simulink model "CCMHfaMotor"  (R2022b) (continued)
== system CCMHfaMotor/HfaMotor_Runnable_sys == (continued)
  ReHvacMotUrgcUnlck_ReHvacMotUrgcUnlck_write : Outport  Port=59
      settings: EnsureOutportIsVirtual=on
  RHFAScheTableReq_Val_write : Outport  Port=60
      settings: EnsureOutportIsVirtual=on
  WtrMotAdr_WtrMotAdr_write : Outport  Port=61
      settings: EnsureOutportIsVirtual=on
  WtrMotBlockCmd_WtrMotBlockCmd_write : Outport  Port=62
      settings: EnsureOutportIsVirtual=on
  WtrMotClearReq_WtrMotClearReq_write : Outport  Port=63
      settings: EnsureOutportIsVirtual=on
  WtrMotCoilPwrOn_WtrMotCoilPwrOn_write : Outport  Port=64
      settings: EnsureOutportIsVirtual=on
  WtrMotDir_WtrMotDir_write : Outport  Port=65
      settings: EnsureOutportIsVirtual=on
  WtrMotMode_WtrMotMode_write : Outport  Port=66
      settings: EnsureOutportIsVirtual=on
  WtrMotPsonAvl_WtrMotPsonAvl_write : Outport  Port=67
      settings: EnsureOutportIsVirtual=on
  WtrMotSaveData_WtrMotSaveData_write : Outport  Port=68
      settings: EnsureOutportIsVirtual=on
  WtrMotSpdSet_WtrMotSpdSet_write : Outport  Port=69
      settings: EnsureOutportIsVirtual=on
  WtrMotStrtPson_WtrMotStrtPson_write : Outport  Port=70
      settings: EnsureOutportIsVirtual=on
  WtrMotTargtPson_WtrMotTargtPson_write : Outport  Port=71
      settings: EnsureOutportIsVirtual=on
  WtrMotUrgcPson_WtrMotUrgcPson_write : Outport  Port=72
      settings: EnsureOutportIsVirtual=on
  WtrMotUrgcUnlck_WtrMotUrgcUnlck_write : Outport  Port=73
      settings: EnsureOutportIsVirtual=on
  HfaMotAdr_HfaMotAdr_write : Outport  Port=74
      settings: EnsureOutportIsVirtual=on
  HfaMotBlockCmd_HfaMotBlockCmd_write : Outport  Port=75
      settings: EnsureOutportIsVirtual=on
  HfaMotClearReq_HfaMotClearReq_write : Outport  Port=76
      settings: EnsureOutportIsVirtual=on
  HfaMotCoilPwrOn_HfaMotCoilPwrOn_write : Outport  Port=77
      settings: EnsureOutportIsVirtual=on
  HfaMotDir_HfaMotDir_write : Outport  Port=78
      settings: EnsureOutportIsVirtual=on
  HfaMotMode_HfaMotMode_write : Outport  Port=79
      settings: EnsureOutportIsVirtual=on
  HfaMotPsonAvl_HfaMotPsonAvl_write : Outport  Port=80
      settings: EnsureOutportIsVirtual=on
  HfaMotSaveData_HfaMotSaveData_write : Outport  Port=81
      settings: EnsureOutportIsVirtual=on
  HfaMotSpdSet_HfaMotSpdSet_write : Outport  Port=82
      settings: EnsureOutportIsVirtual=on
  HfaMotStrtPson_HfaMotStrtPson_write : Outport  Port=83
      settings: EnsureOutportIsVirtual=on
  HfaMotTargtPson_HfaMotTargtPson_write : Outport  Port=84
      settings: EnsureOutportIsVirtual=on
  HfaMotUrgcPson_HfaMotUrgcPson_write : Outport  Port=85
      settings: EnsureOutportIsVirtual=on
  HfaMotUrgcUnlck_HfaMotUrgcUnlck_write : Outport  Port=86
      settings: EnsureOutportIsVirtual=on
  wires:
  AcRfrPrgSafety_AcRfrPrgSafety_read/1 -> Terminator_1/1
  ActRfrIdeGroup_ActRfrIdeGroup_read/1 -> Terminator_2/1
  ActRfrIdeItem_ActRfrIdeItem_read/1 -> Terminator_3/1
  ActRfrIdePN_ActRfrIdePN_read/1 -> Terminator_4/1
  ActRfrIdeSupplier_ActRfrIdeSupplier_read/1 -> Terminator_5/1
  ActRfrIdeVers_ActRfrIdeVers_read/1 -> Terminator_6/1
  ActRfrPrgDiret_ActRfrPrgDiret_read/1 -> Terminator_7/1
  ActRfrPrgGroup_ActRfrPrgGroup_read/1 -> Terminator_8/1
  ActRfrPrgItem_ActRfrPrgItem_read/1 -> Terminator_9/1
  ActRfrPrgPrgGroup_ActRfrPrgPrgGroup_read/1 -> Terminator_10/1
  ActRfrPrgPrgItem_ActRfrPrgPrgItem_read/1 -> Terminator_11/1
  ActRfrPrgRef_ActRfrPrgRef_read/1 -> Terminator_12/1
  ActRfrPrgSafetyEnable_ActRfrPrgSafetyEnable_read/1 -> Terminator_13/1
  ActRfrStaCPos_ActRfrStaCPos_read/1 -> Terminator_14/1
  ActRfrStaEmgyRun_ActRfrStaEmgyRun_read/1 -> Terminator_15/1
  ActRfrStaErr_ActRfrStaErr_read/1 -> Terminator_16/1
  ActRfrStaGroup_ActRfrStaGroup_read/1 -> Terminator_17/1
  ActRfrStaItem_ActRfrStaItem_read/1 -> Terminator_18/1
  ActRfrStaMod_ActRfrStaMod_read/1 -> Terminator_19/1
  ActRfrStaReset_ActRfrStaReset_read/1 -> Terminator_20/1
  ActRfrStaStd_ActRfrStaStd_read/1 -> Terminator_21/1
  BatSysBtrMSts_BatSysBtrMSts_read/1 -> Terminator_22/1
  HfaCcmSlaveRespB0Lin4_HfaCcmSlaveRespB0Lin4_read/1 -> Terminator_23/1
  HfaCcmSlaveRespB1Lin4_HfaCcmSlaveRespB1Lin4_read/1 -> Terminator_24/1
  HfaCcmSlaveRespB2Lin4_HfaCcmSlaveRespB2Lin4_read/1 -> Terminator_25/1
  HfaCcmSlaveRespB3Lin4_HfaCcmSlaveRespB3Lin4_read/1 -> Terminator_26/1
  HfaCcmSlaveRespB4Lin4_HfaCcmSlaveRespB4Lin4_read/1 -> Terminator_27/1
  HfaCcmSlaveRespB5Lin4_HfaCcmSlaveRespB5Lin4_read/1 -> Terminator_28/1
  HfaCcmSlaveRespB6Lin4_HfaCcmSlaveRespB6Lin4_read/1 -> Terminator_29/1
  HfaCcmSlaveRespB7Lin4_HfaCcmSlaveRespB7Lin4_read/1 -> Terminator_30/1
  HmiElecAirSwngReq_HmiElecAirSwngReq_read/1 -> Terminator_31/1
  HvacCfgForClimaCtrl_HvacCfgForHvacFrontBlowerType_read/1 -> Terminator_32/1
  HvacCfgForClimaCtrl_HvacCfgForHvacMiscExistSts_read/1 -> Terminator_33/1
  HvacCfgForClimaCtrl_HvacCfgForHvacMotorExistSts_read/1 -> Terminator_34/1
  HvacCfgForClimaCtrl_HvacCfgForHvacSensorExistSts_read/1 -> Terminator_35/1
  SbcPwrOn_SbcPwrOn_read/1 -> Terminator_36/1
  SbcWakeupSta_SbcWakeupSta_read/1 -> Terminator_37/1
  SysServCarCfgSts_SysServCarID_read/1 -> Terminator_38/1
  SysServCarCfgSts_SysServCCPAQSSts_read/1 -> Terminator_39/1
  SysServCarCfgSts_SysServCCPHEXSensorSts_read/1 -> Terminator_40/1
  SysServCarCfgSts_SysServCCPLin2AFUSts_read/1 -> Terminator_41/1
  SysServCarCfgSts_SysServCCPLin2AIUSts_read/1 -> Terminator_42/1
  SysServCarCfgSts_SysServCCPLin2CDSSts_read/1 -> Terminator_43/1
  SysServCarCfgSts_SysServCCPLin2PMSISts_read/1 -> Terminator_44/1
  SysServCarCfgSts_SysServCCPLin3VGASts_read/1 -> Terminator_45/1
  SysServCarCfgSts_SysServDUAirVentSnrSts_read/1 -> Terminator_46/1
  SysServCarCfgSts_SysServDUBlowerPwmSignSts_read/1 -> Terminator_47/1
  SysServCarCfgSts_SysServDUBlowerPwmSts_read/1 -> Terminator_48/1
  SysServCarCfgSts_SysServDUDCDefrostSts_read/1 -> Terminator_49/1
  SysServCarCfgSts_SysServDUDCModeSts_read/1 -> Terminator_50/1
  SysServCarCfgSts_SysServDUDCRecSts_read/1 -> Terminator_51/1
  SysServCarCfgSts_SysServDUDCSModeSts_read/1 -> Terminator_52/1
  SysServCarCfgSts_SysServDUDCSTLSts_read/1 -> Terminator_53/1
  SysServCarCfgSts_SysServDUDCTLSts_read/1 -> Terminator_54/1
  SysServCarCfgSts_SysServDUDCTRSts_read/1 -> Terminator_55/1
  SysServCarCfgSts_SysServDUFLFootSts_read/1 -> Terminator_56/1
  SysServCarCfgSts_SysServDUFLVentSts_read/1 -> Terminator_57/1
  SysServCarCfgSts_SysServDUFRFootSts_read/1 -> Terminator_58/1
  SysServCarCfgSts_SysServDUFRVentSts_read/1 -> Terminator_59/1
  SysServCarCfgSts_SysServDUIncarMotorSts_read/1 -> Terminator_60/1
  SysServCarCfgSts_SysServDULin1HBMFSts_read/1 -> Terminator_61/1
  SysServCarCfgSts_SysServDULin4HFAFSts_read/1 -> Terminator_62/1
  SysServCarCfgSts_SysServDUSFLFootSts_read/1 -> Terminator_63/1
  SysServCarCfgSts_SysServDUSFLVentSts_read/1 -> Terminator_64/1
  SysServCarCfgSts_SysServDUSFRFootSts_read/1 -> Terminator_65/1
  SysServCarCfgSts_SysServDUSFRVentSts_read/1 -> Terminator_66/1
  SysServCarCfgSts_SysServDUSnsrForFCmptmtTSts_read/1 -> Terminator_67/1
  SysServCarCfgSts_SysServDUSnsrForRair_read/1 -> Terminator_68/1
  SysServCarCfgSts_SysServDUSnsrForRCmptmtTSts_read/1 -> Terminator_69/1
  BatLoadBtrMSts_BatLoadBtrMSts_read/1 -> Terminator_70/1
  HfaMotSetPowerOnInit_PowerOnInit_read/1 -> Terminator_71/1
  VehModMngtGlbSafe1_VehModMngtGlbSafe1_read/1 -> Terminator_72/1
  HfaActr01ActPson_HfaActr01ActPson_read/1 -> Terminator_73/1
  HfaActr01ActPson_HfaActr01ActPson_IsUpdated_read/1 -> Terminator_74/1
  HfaActr01BlckInd_HfaActr01BlckInd_read/1 -> Terminator_75/1
  HfaActr01BlckSts_HfaActr01BlckSts_read/1 -> Terminator_76/1
  HfaActr01BusAdr_HfaActr01BusAdr_read/1 -> Terminator_77/1
  HfaActr01CoilSts_HfaActr01CoilSts_read/1 -> Terminator_78/1
  HfaActr01DrvDir_HfaActr01DrvDir_read/1 -> Terminator_79/1
  HfaActr01ElecErr_HfaActr01ElecErr_read/1 -> Terminator_80/1
  HfaActr01Mode_HfaActr01Mode_read/1 -> Terminator_81/1
  HfaActr01MovDir_HfaActr01MovDir_read/1 -> Terminator_82/1
  HfaActr01OvrTempErr_HfaActr01OvrTempErr_read/1 -> Terminator_83/1
  HfaActr01PsonSts_HfaActr01PsonSts_read/1 -> Terminator_84/1
  HfaActr01ResetSts_HfaActr01ResetSts_read/1 -> Terminator_85/1
  HfaActr01SpclFct_HfaActr01SpclFct_read/1 -> Terminator_86/1
  HfaActr01SpdSts_HfaActr01SpdSts_read/1 -> Terminator_87/1
  HfaActr01TrqSts_HfaActr01TrqSts_read/1 -> Terminator_88/1
  HfaActr01UrgcExist_HfaActr01UrgcExist_read/1 -> Terminator_89/1
  HfaActr01UrgcPson_HfaActr01UrgcPson_read/1 -> Terminator_90/1
  HfaActr01UrgcSts_HfaActr01UrgcSts_read/1 -> Terminator_91/1
  HfaActr01VoltErr_HfaActr01VoltErr_read/1 -> Terminator_92/1
  HfaActr02ActPson_HfaActr02ActPson_read/1 -> Terminator_93/1
  HfaActr02ActPson_HfaActr02ActPson_IsUpdated_read/1 -> Terminator_94/1
  HfaActr02BlckInd_HfaActr02BlckInd_read/1 -> Terminator_95/1
  HfaActr02BlckSts_HfaActr02BlckSts_read/1 -> Terminator_96/1
  HfaActr02BusAdr_HfaActr02BusAdr_read/1 -> Terminator_97/1
  HfaActr02CoilSts_HfaActr02CoilSts_read/1 -> Terminator_98/1
  HfaActr02DrvDir_HfaActr02DrvDir_read/1 -> Terminator_99/1
  HfaActr02ElecErr_HfaActr02ElecErr_read/1 -> Terminator_100/1
  HfaActr02Mode_HfaActr02Mode_read/1 -> Terminator_101/1
  HfaActr02MovDir_HfaActr02MovDir_read/1 -> Terminator_102/1
  HfaActr02OvrTempErr_HfaActr02OvrTempErr_read/1 -> Terminator_103/1
  HfaActr02PsonSts_HfaActr02PsonSts_read/1 -> Terminator_104/1
  HfaActr02ResetSts_HfaActr02ResetSts_read/1 -> Terminator_105/1
  HfaActr02SpclFct_HfaActr02SpclFct_read/1 -> Terminator_106/1
  HfaActr02SpdSts_HfaActr02SpdSts_read/1 -> Terminator_107/1
  HfaActr02TrqSts_HfaActr02TrqSts_read/1 -> Terminator_108/1
  HfaActr02UrgcExist_HfaActr02UrgcExist_read/1 -> Terminator_109/1
  HfaActr02UrgcPson_HfaActr02UrgcPson_read/1 -> Terminator_110/1
  HfaActr02UrgcSts_HfaActr02UrgcSts_read/1 -> Terminator_111/1
  HfaActr02VoltErr_HfaActr02VoltErr_read/1 -> Terminator_112/1
  HfaActr03ActPson_HfaActr03ActPson_read/1 -> Terminator_113/1
  HfaActr03ActPson_HfaActr03ActPson_IsUpdated_read/1 -> Terminator_114/1
  HfaActr03BlckInd_HfaActr03BlckInd_read/1 -> Terminator_115/1
  HfaActr03BlckSts_HfaActr03BlckSts_read/1 -> Terminator_116/1
  HfaActr03BusAdr_HfaActr03BusAdr_read/1 -> Terminator_117/1
  HfaActr03CoilSts_HfaActr03CoilSts_read/1 -> Terminator_118/1
  HfaActr03DrvDir_HfaActr03DrvDir_read/1 -> Terminator_119/1
  HfaActr03ElecErr_HfaActr03ElecErr_read/1 -> Terminator_120/1
  HfaActr03Mode_HfaActr03Mode_read/1 -> Terminator_121/1
  HfaActr03MovDir_HfaActr03MovDir_read/1 -> Terminator_122/1
  HfaActr03OvrTempErr_HfaActr03OvrTempErr_read/1 -> Terminator_123/1
  HfaActr03PsonSts_HfaActr03PsonSts_read/1 -> Terminator_124/1
  HfaActr03ResetSts_HfaActr03ResetSts_read/1 -> Terminator_125/1
  HfaActr03SpclFct_HfaActr03SpclFct_read/1 -> Terminator_126/1
  HfaActr03SpdSts_HfaActr03SpdSts_read/1 -> Terminator_127/1
  HfaActr03TrqSts_HfaActr03TrqSts_read/1 -> Terminator_128/1
  HfaActr03UrgcExist_HfaActr03UrgcExist_read/1 -> Terminator_129/1
  HfaActr03UrgcPson_HfaActr03UrgcPson_read/1 -> Terminator_130/1
  HfaActr03UrgcSts_HfaActr03UrgcSts_read/1 -> Terminator_131/1
  HfaActr03VoltErr_HfaActr03VoltErr_read/1 -> Terminator_132/1
  HfaActr04ActPson_HfaActr04ActPson_read/1 -> Terminator_133/1
  HfaActr04ActPson_HfaActr04ActPson_IsUpdated_read/1 -> Terminator_134/1
  HfaActr04BlckInd_HfaActr04BlckInd_read/1 -> Terminator_135/1
  HfaActr04BlckSts_HfaActr04BlckSts_read/1 -> Terminator_136/1
  HfaActr04BusAdr_HfaActr04BusAdr_read/1 -> Terminator_137/1
  HfaActr04CoilSts_HfaActr04CoilSts_read/1 -> Terminator_138/1
  HfaActr04DrvDir_HfaActr04DrvDir_read/1 -> Terminator_139/1
  HfaActr04ElecErr_HfaActr04ElecErr_read/1 -> Terminator_140/1
  HfaActr04Mode_HfaActr04Mode_read/1 -> Terminator_141/1
  HfaActr04MovDir_HfaActr04MovDir_read/1 -> Terminator_142/1
  HfaActr04OvrTempErr_HfaActr04OvrTempErr_read/1 -> Terminator_143/1
  HfaActr04PsonSts_HfaActr04PsonSts_read/1 -> Terminator_144/1
  HfaActr04ResetSts_HfaActr04ResetSts_read/1 -> Terminator_145/1
  HfaActr04SpclFct_HfaActr04SpclFct_read/1 -> Terminator_146/1
  HfaActr04SpdSts_HfaActr04SpdSts_read/1 -> Terminator_147/1
  HfaActr04TrqSts_HfaActr04TrqSts_read/1 -> Terminator_148/1
  HfaActr04UrgcExist_HfaActr04UrgcExist_read/1 -> Terminator_149/1
  HfaActr04UrgcPson_HfaActr04UrgcPson_read/1 -> Terminator_150/1
  HfaActr04UrgcSts_HfaActr04UrgcSts_read/1 -> Terminator_151/1
  HfaActr04VoltErr_HfaActr04VoltErr_read/1 -> Terminator_152/1
  HfaActr05ActPson_HfaActr05ActPson_read/1 -> Terminator_153/1
  HfaActr05ActPson_HfaActr05ActPson_IsUpdated_read/1 -> Terminator_154/1
  HfaActr05BlckInd_HfaActr05BlckInd_read/1 -> Terminator_155/1
  HfaActr05BlckSts_HfaActr05BlckSts_read/1 -> Terminator_156/1
  HfaActr05BusAdr_HfaActr05BusAdr_read/1 -> Terminator_157/1
  HfaActr05CoilSts_HfaActr05CoilSts_read/1 -> Terminator_158/1
  HfaActr05DrvDir_HfaActr05DrvDir_read/1 -> Terminator_159/1
  HfaActr05ElecErr_HfaActr05ElecErr_read/1 -> Terminator_160/1
  HfaActr05Mode_HfaActr05Mode_read/1 -> Terminator_161/1
  HfaActr05MovDir_HfaActr05MovDir_read/1 -> Terminator_162/1
  HfaActr05OvrTempErr_HfaActr05OvrTempErr_read/1 -> Terminator_163/1
  HfaActr05PsonSts_HfaActr05PsonSts_read/1 -> Terminator_164/1
  HfaActr05ResetSts_HfaActr05ResetSts_read/1 -> Terminator_165/1
  HfaActr05SpclFct_HfaActr05SpclFct_read/1 -> Terminator_166/1
  HfaActr05SpdSts_HfaActr05SpdSts_read/1 -> Terminator_167/1
  HfaActr05TrqSts_HfaActr05TrqSts_read/1 -> Terminator_168/1
  HfaActr05UrgcExist_HfaActr05UrgcExist_read/1 -> Terminator_169/1
  HfaActr05UrgcPson_HfaActr05UrgcPson_read/1 -> Terminator_170/1
  HfaActr05UrgcSts_HfaActr05UrgcSts_read/1 -> Terminator_171/1
  HfaActr05VoltErr_HfaActr05VoltErr_read/1 -> Terminator_172/1
  HfaActr06ActPson_HfaActr06ActPson_read/1 -> Terminator_173/1
  HfaActr06ActPson_HfaActr06ActPson_IsUpdated_read/1 -> Terminator_174/1
  HfaActr06BlckInd_HfaActr06BlckInd_read/1 -> Terminator_175/1
  HfaActr06BlckSts_HfaActr06BlckSts_read/1 -> Terminator_176/1
  HfaActr06BusAdr_HfaActr06BusAdr_read/1 -> Terminator_177/1
  HfaActr06CoilSts_HfaActr06CoilSts_read/1 -> Terminator_178/1
  HfaActr06DrvDir_HfaActr06DrvDir_read/1 -> Terminator_179/1
  HfaActr06ElecErr_HfaActr06ElecErr_read/1 -> Terminator_180/1
  HfaActr06Mode_HfaActr06Mode_read/1 -> Terminator_181/1
  HfaActr06MovDir_HfaActr06MovDir_read/1 -> Terminator_182/1
  HfaActr06OvrTempErr_HfaActr06OvrTempErr_read/1 -> Terminator_183/1
  HfaActr06PsonSts_HfaActr06PsonSts_read/1 -> Terminator_184/1
  HfaActr06ResetSts_HfaActr06ResetSts_read/1 -> Terminator_185/1
  HfaActr06SpclFct_HfaActr06SpclFct_read/1 -> Terminator_186/1
  HfaActr06SpdSts_HfaActr06SpdSts_read/1 -> Terminator_187/1
  HfaActr06TrqSts_HfaActr06TrqSts_read/1 -> Terminator_188/1
  HfaActr06UrgcExist_HfaActr06UrgcExist_read/1 -> Terminator_189/1
  HfaActr06UrgcPson_HfaActr06UrgcPson_read/1 -> Terminator_190/1
  HfaActr06UrgcSts_HfaActr06UrgcSts_read/1 -> Terminator_191/1
  HfaActr06VoltErr_HfaActr06VoltErr_read/1 -> Terminator_192/1
  HfaActr07ActPson_HfaActr07ActPson_read/1 -> Terminator_193/1
  HfaActr07ActPson_HfaActr07ActPson_IsUpdated_read/1 -> Terminator_194/1
  HfaActr07BlckInd_HfaActr07BlckInd_read/1 -> Terminator_195/1
  HfaActr07BlckSts_HfaActr07BlckSts_read/1 -> Terminator_196/1
  HfaActr07BusAdr_HfaActr07BusAdr_read/1 -> Terminator_197/1
  HfaActr07CoilSts_HfaActr07CoilSts_read/1 -> Terminator_198/1
  HfaActr07DrvDir_HfaActr07DrvDir_read/1 -> Terminator_199/1
  HfaActr07ElecErr_HfaActr07ElecErr_read/1 -> Terminator_200/1
  HfaActr07Mode_HfaActr07Mode_read/1 -> Terminator_201/1
  HfaActr07MovDir_HfaActr07MovDir_read/1 -> Terminator_202/1
  HfaActr07OvrTempErr_HfaActr07OvrTempErr_read/1 -> Terminator_203/1
  HfaActr07PsonSts_HfaActr07PsonSts_read/1 -> Terminator_204/1
  HfaActr07ResetSts_HfaActr07ResetSts_read/1 -> Terminator_205/1
  HfaActr07SpclFct_HfaActr07SpclFct_read/1 -> Terminator_206/1
  HfaActr07SpdSts_HfaActr07SpdSts_read/1 -> Terminator_207/1
  HfaActr07TrqSts_HfaActr07TrqSts_read/1 -> Terminator_208/1
  HfaActr07UrgcExist_HfaActr07UrgcExist_read/1 -> Terminator_209/1
  HfaActr07UrgcPson_HfaActr07UrgcPson_read/1 -> Terminator_210/1
  HfaActr07UrgcSts_HfaActr07UrgcSts_read/1 -> Terminator_211/1
  HfaActr07VoltErr_HfaActr07VoltErr_read/1 -> Terminator_212/1
  HfaActr08ActPson_HfaActr08ActPson_read/1 -> Terminator_213/1
  HfaActr08ActPson_HfaActr08ActPson_IsUpdated_read/1 -> Terminator_214/1
  HfaActr08BlckInd_HfaActr08BlckInd_read/1 -> Terminator_215/1
  HfaActr08BlckSts_HfaActr08BlckSts_read/1 -> Terminator_216/1
  HfaActr08BusAdr_HfaActr08BusAdr_read/1 -> Terminator_217/1
  HfaActr08CoilSts_HfaActr08CoilSts_read/1 -> Terminator_218/1
  HfaActr08DrvDir_HfaActr08DrvDir_read/1 -> Terminator_219/1
  HfaActr08ElecErr_HfaActr08ElecErr_read/1 -> Terminator_220/1
  HfaActr08Mode_HfaActr08Mode_read/1 -> Terminator_221/1
  HfaActr08MovDir_HfaActr08MovDir_read/1 -> Terminator_222/1
  HfaActr08OvrTempErr_HfaActr08OvrTempErr_read/1 -> Terminator_223/1
  HfaActr08PsonSts_HfaActr08PsonSts_read/1 -> Terminator_224/1
  HfaActr08ResetSts_HfaActr08ResetSts_read/1 -> Terminator_225/1
  HfaActr08SpclFct_HfaActr08SpclFct_read/1 -> Terminator_226/1
  HfaActr08SpdSts_HfaActr08SpdSts_read/1 -> Terminator_227/1
  HfaActr08TrqSts_HfaActr08TrqSts_read/1 -> Terminator_228/1
  HfaActr08UrgcExist_HfaActr08UrgcExist_read/1 -> Terminator_229/1
  HfaActr08UrgcPson_HfaActr08UrgcPson_read/1 -> Terminator_230/1
  HfaActr08UrgcSts_HfaActr08UrgcSts_read/1 -> Terminator_231/1
  HfaActr08VoltErr_HfaActr08VoltErr_read/1 -> Terminator_232/1
  HfaActr09ActPson_HfaActr09ActPson_read/1 -> Terminator_233/1
  HfaActr09ActPson_HfaActr09ActPson_IsUpdated_read/1 -> Terminator_234/1
  HfaActr09BlckInd_HfaActr09BlckInd_read/1 -> Terminator_235/1
  HfaActr09BlckSts_HfaActr09BlckSts_read/1 -> Terminator_236/1
  HfaActr09BusAdr_HfaActr09BusAdr_read/1 -> Terminator_237/1
  HfaActr09CoilSts_HfaActr09CoilSts_read/1 -> Terminator_238/1
  HfaActr09DrvDir_HfaActr09DrvDir_read/1 -> Terminator_239/1
  HfaActr09ElecErr_HfaActr09ElecErr_read/1 -> Terminator_240/1
  HfaActr09Mode_HfaActr09Mode_read/1 -> Terminator_241/1
  HfaActr09MovDir_HfaActr09MovDir_read/1 -> Terminator_242/1
  HfaActr09OvrTempErr_HfaActr09OvrTempErr_read/1 -> Terminator_243/1
  HfaActr09PsonSts_HfaActr09PsonSts_read/1 -> Terminator_244/1
  HfaActr09ResetSts_HfaActr09ResetSts_read/1 -> Terminator_245/1
  HfaActr09SpclFct_HfaActr09SpclFct_read/1 -> Terminator_246/1
  HfaActr09SpdSts_HfaActr09SpdSts_read/1 -> Terminator_247/1
  HfaActr09TrqSts_HfaActr09TrqSts_read/1 -> Terminator_248/1
  HfaActr09UrgcExist_HfaActr09UrgcExist_read/1 -> Terminator_249/1
  HfaActr09UrgcPson_HfaActr09UrgcPson_read/1 -> Terminator_250/1
  HfaActr09UrgcSts_HfaActr09UrgcSts_read/1 -> Terminator_251/1
  HfaActr09VoltErr_HfaActr09VoltErr_read/1 -> Terminator_252/1
  HfaActr10ActPson_HfaActr10ActPson_read/1 -> Terminator_253/1
  HfaActr10ActPson_HfaActr10ActPson_IsUpdated_read/1 -> Terminator_254/1
  HfaActr10BlckInd_HfaActr10BlckInd_read/1 -> Terminator_255/1
  HfaActr10BlckSts_HfaActr10BlckSts_read/1 -> Terminator_256/1
  HfaActr10BusAdr_HfaActr10BusAdr_read/1 -> Terminator_257/1
  HfaActr10CoilSts_HfaActr10CoilSts_read/1 -> Terminator_258/1
  HfaActr10DrvDir_HfaActr10DrvDir_read/1 -> Terminator_259/1
  HfaActr10ElecErr_HfaActr10ElecErr_read/1 -> Terminator_260/1
  HfaActr10Mode_HfaActr10Mode_read/1 -> Terminator_261/1
  HfaActr10MovDir_HfaActr10MovDir_read/1 -> Terminator_262/1
  HfaActr10OvrTempErr_HfaActr10OvrTempErr_read/1 -> Terminator_263/1
  HfaActr10PsonSts_HfaActr10PsonSts_read/1 -> Terminator_264/1
  HfaActr10ResetSts_HfaActr10ResetSts_read/1 -> Terminator_265/1
  HfaActr10SpclFct_HfaActr10SpclFct_read/1 -> Terminator_266/1
  HfaActr10SpdSts_HfaActr10SpdSts_read/1 -> Terminator_267/1
  HfaActr10TrqSts_HfaActr10TrqSts_read/1 -> Terminator_268/1
  HfaActr10UrgcExist_HfaActr10UrgcExist_read/1 -> Terminator_269/1
  HfaActr10UrgcPson_HfaActr10UrgcPson_read/1 -> Terminator_270/1
  HfaActr10UrgcSts_HfaActr10UrgcSts_read/1 -> Terminator_271/1
  HfaActr10VoltErr_HfaActr10VoltErr_read/1 -> Terminator_272/1
  HfaActr11ActPson_HfaActr11ActPson_read/1 -> Terminator_273/1
  HfaActr11ActPson_HfaActr11ActPson_IsUpdated_read/1 -> Terminator_274/1
  HfaActr11BlckInd_HfaActr11BlckInd_read/1 -> Terminator_275/1
  HfaActr11BlckSts_HfaActr11BlckSts_read/1 -> Terminator_276/1
  HfaActr11BusAdr_HfaActr11BusAdr_read/1 -> Terminator_277/1
  HfaActr11CoilSts_HfaActr11CoilSts_read/1 -> Terminator_278/1
  HfaActr11DrvDir_HfaActr11DrvDir_read/1 -> Terminator_279/1
  HfaActr11ElecErr_HfaActr11ElecErr_read/1 -> Terminator_280/1
  HfaActr11Mode_HfaActr11Mode_read/1 -> Terminator_281/1
  HfaActr11MovDir_HfaActr11MovDir_read/1 -> Terminator_282/1
  HfaActr11OvrTempErr_HfaActr11OvrTempErr_read/1 -> Terminator_283/1
  HfaActr11PsonSts_HfaActr11PsonSts_read/1 -> Terminator_284/1
  HfaActr11ResetSts_HfaActr11ResetSts_read/1 -> Terminator_285/1
  HfaActr11SpclFct_HfaActr11SpclFct_read/1 -> Terminator_286/1
  HfaActr11SpdSts_HfaActr11SpdSts_read/1 -> Terminator_287/1
  HfaActr11TrqSts_HfaActr11TrqSts_read/1 -> Terminator_288/1
  HfaActr11UrgcExist_HfaActr11UrgcExist_read/1 -> Terminator_289/1
  HfaActr11UrgcPson_HfaActr11UrgcPson_read/1 -> Terminator_290/1
  HfaActr11UrgcSts_HfaActr11UrgcSts_read/1 -> Terminator_291/1
  HfaActr11VoltErr_HfaActr11VoltErr_read/1 -> Terminator_292/1
  HfaActr12ActPson_HfaActr12ActPson_read/1 -> Terminator_293/1
  HfaActr12ActPson_HfaActr12ActPson_IsUpdated_read/1 -> Terminator_294/1
  HfaActr12BlckInd_HfaActr12BlckInd_read/1 -> Terminator_295/1
  HfaActr12BlckSts_HfaActr12BlckSts_read/1 -> Terminator_296/1
  HfaActr12BusAdr_HfaActr12BusAdr_read/1 -> Terminator_297/1
  HfaActr12CoilSts_HfaActr12CoilSts_read/1 -> Terminator_298/1
  HfaActr12DrvDir_HfaActr12DrvDir_read/1 -> Terminator_299/1
  HfaActr12ElecErr_HfaActr12ElecErr_read/1 -> Terminator_300/1
  HfaActr12Mode_HfaActr12Mode_read/1 -> Terminator_301/1
  HfaActr12MovDir_HfaActr12MovDir_read/1 -> Terminator_302/1
  HfaActr12OvrTempErr_HfaActr12OvrTempErr_read/1 -> Terminator_303/1
  HfaActr12PsonSts_HfaActr12PsonSts_read/1 -> Terminator_304/1
  HfaActr12ResetSts_HfaActr12ResetSts_read/1 -> Terminator_305/1
  HfaActr12SpclFct_HfaActr12SpclFct_read/1 -> Terminator_306/1
  HfaActr12SpdSts_HfaActr12SpdSts_read/1 -> Terminator_307/1
  HfaActr12TrqSts_HfaActr12TrqSts_read/1 -> Terminator_308/1
  HfaActr12UrgcExist_HfaActr12UrgcExist_read/1 -> Terminator_309/1
  HfaActr12UrgcPson_HfaActr12UrgcPson_read/1 -> Terminator_310/1
  HfaActr12UrgcSts_HfaActr12UrgcSts_read/1 -> Terminator_311/1
  HfaActr12VoltErr_HfaActr12VoltErr_read/1 -> Terminator_312/1
  HfaActr13ActPson_HfaActr13ActPson_read/1 -> Terminator_313/1
  HfaActr13ActPson_HfaActr13ActPson_IsUpdated_read/1 -> Terminator_314/1
  HfaActr13BlckInd_HfaActr13BlckInd_read/1 -> Terminator_315/1
  HfaActr13BlckSts_HfaActr13BlckSts_read/1 -> Terminator_316/1
  HfaActr13BusAdr_HfaActr13BusAdr_read/1 -> Terminator_317/1
  HfaActr13CoilSts_HfaActr13CoilSts_read/1 -> Terminator_318/1
  HfaActr13DrvDir_HfaActr13DrvDir_read/1 -> Terminator_319/1
  HfaActr13ElecErr_HfaActr13ElecErr_read/1 -> Terminator_320/1
  HfaActr13Mode_HfaActr13Mode_read/1 -> Terminator_321/1
  HfaActr13MovDir_HfaActr13MovDir_read/1 -> Terminator_322/1
  HfaActr13OvrTempErr_HfaActr13OvrTempErr_read/1 -> Terminator_323/1
  HfaActr13PsonSts_HfaActr13PsonSts_read/1 -> Terminator_324/1
  HfaActr13ResetSts_HfaActr13ResetSts_read/1 -> Terminator_325/1
  HfaActr13SpclFct_HfaActr13SpclFct_read/1 -> Terminator_326/1
  HfaActr13SpdSts_HfaActr13SpdSts_read/1 -> Terminator_327/1
  HfaActr13TrqSts_HfaActr13TrqSts_read/1 -> Terminator_328/1
  HfaActr13UrgcExist_HfaActr13UrgcExist_read/1 -> Terminator_329/1
  HfaActr13UrgcPson_HfaActr13UrgcPson_read/1 -> Terminator_330/1
  HfaActr13UrgcSts_HfaActr13UrgcSts_read/1 -> Terminator_331/1
  HfaActr13VoltErr_HfaActr13VoltErr_read/1 -> Terminator_332/1
  HfaActr14ActPson_HfaActr14ActPson_read/1 -> Terminator_333/1
  HfaActr14ActPson_HfaActr14ActPson_IsUpdated_read/1 -> Terminator_334/1
  HfaActr14BlckInd_HfaActr14BlckInd_read/1 -> Terminator_335/1
  HfaActr14BlckSts_HfaActr14BlckSts_read/1 -> Terminator_336/1
  HfaActr14BusAdr_HfaActr14BusAdr_read/1 -> Terminator_337/1
  HfaActr14CoilSts_HfaActr14CoilSts_read/1 -> Terminator_338/1
  HfaActr14DrvDir_HfaActr14DrvDir_read/1 -> Terminator_339/1
  HfaActr14ElecErr_HfaActr14ElecErr_read/1 -> Terminator_340/1
  HfaActr14Mode_HfaActr14Mode_read/1 -> Terminator_341/1
  HfaActr14MovDir_HfaActr14MovDir_read/1 -> Terminator_342/1
  HfaActr14OvrTempErr_HfaActr14OvrTempErr_read/1 -> Terminator_343/1
  HfaActr14PsonSts_HfaActr14PsonSts_read/1 -> Terminator_344/1
  HfaActr14ResetSts_HfaActr14ResetSts_read/1 -> Terminator_345/1
  HfaActr14SpclFct_HfaActr14SpclFct_read/1 -> Terminator_346/1
  HfaActr14SpdSts_HfaActr14SpdSts_read/1 -> Terminator_347/1
  HfaActr14TrqSts_HfaActr14TrqSts_read/1 -> Terminator_348/1
  HfaActr14UrgcExist_HfaActr14UrgcExist_read/1 -> Terminator_349/1
  HfaActr14UrgcPson_HfaActr14UrgcPson_read/1 -> Terminator_350/1
  HfaActr14UrgcSts_HfaActr14UrgcSts_read/1 -> Terminator_351/1
  HfaActr14VoltErr_HfaActr14VoltErr_read/1 -> Terminator_352/1
  HfaActr15ActPson_HfaActr15ActPson_read/1 -> Terminator_353/1
  HfaActr15ActPson_HfaActr15ActPson_IsUpdated_read/1 -> Terminator_354/1
  HfaActr15BlckInd_HfaActr15BlckInd_read/1 -> Terminator_355/1
  HfaActr15BlckSts_HfaActr15BlckSts_read/1 -> Terminator_356/1
  HfaActr15BusAdr_HfaActr15BusAdr_read/1 -> Terminator_357/1
  HfaActr15CoilSts_HfaActr15CoilSts_read/1 -> Terminator_358/1
  HfaActr15DrvDir_HfaActr15DrvDir_read/1 -> Terminator_359/1
  HfaActr15ElecErr_HfaActr15ElecErr_read/1 -> Terminator_360/1
  HfaActr15Mode_HfaActr15Mode_read/1 -> Terminator_361/1
  HfaActr15MovDir_HfaActr15MovDir_read/1 -> Terminator_362/1
  HfaActr15OvrTempErr_HfaActr15OvrTempErr_read/1 -> Terminator_363/1
  HfaActr15PsonSts_HfaActr15PsonSts_read/1 -> Terminator_364/1
  HfaActr15ResetSts_HfaActr15ResetSts_read/1 -> Terminator_365/1
  HfaActr15SpclFct_HfaActr15SpclFct_read/1 -> Terminator_366/1
  HfaActr15SpdSts_HfaActr15SpdSts_read/1 -> Terminator_367/1
  HfaActr15TrqSts_HfaActr15TrqSts_read/1 -> Terminator_368/1
  HfaActr15UrgcExist_HfaActr15UrgcExist_read/1 -> Terminator_369/1
  HfaActr15UrgcPson_HfaActr15UrgcPson_read/1 -> Terminator_370/1
  HfaActr15UrgcSts_HfaActr15UrgcSts_read/1 -> Terminator_371/1
  HfaActr15VoltErr_HfaActr15VoltErr_read/1 -> Terminator_372/1
  HvacCfgForClimaCtrl_HvacCfgForAQSTypes_read/1 -> Terminator_373/1
  HvacCfgForClimaCtrl_HvacCfgForHvacLowSideRETXVESts_read/1 -> Terminator_374/1
  HvacCfgForClimaCtrl_HvacCfgForHvacReBlowerType_read/1 -> Terminator_375/1
  HvacCfgForClimaCtrl_HvacCfgForHvacReVentPstn_read/1 -> Terminator_376/1
  HvacCfgForClimaCtrl_HvacCfgForHvacSnsrRCmptmtESts_read/1 -> Terminator_377/1
  HvacCfgForClimaCtrl_HvacCfgForHvacSnsrReHexESts_read/1 -> Terminator_378/1
  Lin1ActvReady_Lin1ActvReady_read/1 -> Terminator_379/1
  Lin2ActvReady_Lin2ActvReady_read/1 -> Terminator_380/1
  ReHvacActrActPson_ReHvacActrActPson_read/1 -> Terminator_381/1
  ReHvacActrActPson_ReHvacActrActPson_IsUpdated_read/1 -> Terminator_382/1
  ReHvacActrBlckInd_ReHvacActrBlckInd_read/1 -> Terminator_383/1
  ReHvacActrBlckSts_ReHvacActrBlckSts_read/1 -> Terminator_384/1
  ReHvacActrBusAdr_ReHvacActrBusAdr_read/1 -> Terminator_385/1
  ReHvacActrCoilSts_ReHvacActrCoilSts_read/1 -> Terminator_386/1
  ReHvacActrDrvDir_ReHvacActrDrvDir_read/1 -> Terminator_387/1
  ReHvacActrElecErr_ReHvacActrElecErr_read/1 -> Terminator_388/1
  ReHvacActrMode_ReHvacActrMode_read/1 -> Terminator_389/1
  ReHvacActrMovDir_ReHvacActrMovDir_read/1 -> Terminator_390/1
  ReHvacActrOvrTempErr_ReHvacActrOvrTempErr_read/1 -> Terminator_391/1
  ReHvacActrPsonSts_ReHvacActrPsonSts_read/1 -> Terminator_392/1
  ReHvacActrResetSts_ReHvacActrResetSts_read/1 -> Terminator_393/1
  ReHvacActrSpclFct_ReHvacActrSpclFct_read/1 -> Terminator_394/1
  ReHvacActrSpdSts_ReHvacActrSpdSts_read/1 -> Terminator_395/1
  ReHvacActrTrqSts_ReHvacActrTrqSts_read/1 -> Terminator_396/1
  ReHvacActrUrgcExist_ReHvacActrUrgcExist_read/1 -> Terminator_397/1
  ReHvacActrUrgcPson_ReHvacActrUrgcPson_read/1 -> Terminator_398/1
  ReHvacActrUrgcSts_ReHvacActrUrgcSts_read/1 -> Terminator_399/1
  ReHvacActrVoltErr_ReHvacActrVoltErr_read/1 -> Terminator_400/1
  SysServCarCfgSts_SysServCCPBGMNum_read/1 -> Terminator_401/1
  SysServCarCfgSts_SysServCCPLin2AQSSts_read/1 -> Terminator_402/1
  SysServCarCfgSts_SysServDUBlowerPwmSignTypeSts_read/1 -> Terminator_403/1
  SysServCarCfgSts_SysServDUDCFLMdeSts_read/1 -> Terminator_404/1
  SysServCarCfgSts_SysServDULin4HFAFStsH_read/1 -> Terminator_405/1
  SysServCarCfgSts_SysServDUReBlowerPwmSignSts_read/1 -> Terminator_406/1
  SysServCarCfgSts_SysServDUReBlowerPwmSts_read/1 -> Terminator_407/1
  SysServCarCfgSts_SysServFloatingMotorSts_read/1 -> Terminator_408/1
  SysServCarCfgSts_SysServFrntHEXSnsrPosn_read/1 -> Terminator_409/1
  SysServCarCfgSts_SysServLowSideRETXVSts_read/1 -> Terminator_410/1
  SysServCarCfgSts_SysServReHEXSnsrPosn_read/1 -> Terminator_411/1
  SysServCarCfgSts_SysServRHFASts_read/1 -> Terminator_412/1
  SysServCarCfgSts_SysServSnsrForFCmptmtTType_read/1 -> Terminator_413/1
  WtrActrLeActPson_WtrActrLeActPson_read/1 -> Terminator_414/1
  WtrActrLeBlckInd_WtrActrLeBlckInd_read/1 -> Terminator_415/1
  WtrActrLeBlckSts_WtrActrLeBlckSts_read/1 -> Terminator_416/1
  WtrActrLeBusAdr_WtrActrLeBusAdr_read/1 -> Terminator_417/1
  WtrActrLeCoilSts_WtrActrLeCoilSts_read/1 -> Terminator_418/1
  WtrActrLeDir_WtrActrLeDir_read/1 -> Terminator_419/1
  WtrActrLeElecErr_WtrActrLeElecErr_read/1 -> Terminator_420/1
  WtrActrLeMode_WtrActrLeMode_read/1 -> Terminator_421/1
  WtrActrLeMovDir_WtrActrLeMovDir_read/1 -> Terminator_422/1
  WtrActrLeOvrTempErr_WtrActrLeOvrTempErr_read/1 -> Terminator_423/1
  WtrActrLePsonSts_WtrActrLePsonSts_read/1 -> Terminator_424/1
  WtrActrLeResetSts_WtrActrLeResetSts_read/1 -> Terminator_425/1
  WtrActrLeSpclFct_WtrActrLeSpclFct_read/1 -> Terminator_426/1
  WtrActrLeSpdSts_WtrActrLeSpdSts_read/1 -> Terminator_427/1
  WtrActrLeTrqSts_WtrActrLeTrqSts_read/1 -> Terminator_428/1
  WtrActrLeUrgcExist_WtrActrLeUrgcExist_read/1 -> Terminator_429/1
  WtrActrLeUrgcPson_WtrActrLeUrgcPson_read/1 -> Terminator_430/1
  WtrActrLeUrgcSts_WtrActrLeUrgcSts_read/1 -> Terminator_431/1
  WtrActrLeVoltErr_WtrActrLeVoltErr_read/1 -> Terminator_432/1
  WtrActrRiActPson_WtrActrRiActPson_read/1 -> Terminator_433/1
  WtrActrRiBlckInd_WtrActrRiBlckInd_read/1 -> Terminator_434/1
  WtrActrRiBlckSts_WtrActrRiBlckSts_read/1 -> Terminator_435/1
  WtrActrRiBusAdr_WtrActrRiBusAdr_read/1 -> Terminator_436/1
  WtrActrRiCoilSts_WtrActrRiCoilSts_read/1 -> Terminator_437/1
  WtrActrRiDir_WtrActrRiDir_read/1 -> Terminator_438/1
  WtrActrRiElecErr_WtrActrRiElecErr_read/1 -> Terminator_439/1
  WtrActrRiMode_WtrActrRiMode_read/1 -> Terminator_440/1
  WtrActrRiMovDir_WtrActrRiMovDir_read/1 -> Terminator_441/1
  WtrActrRiOvrTempErr_WtrActrRiOvrTempErr_read/1 -> Terminator_442/1
  WtrActrRiPsonSts_WtrActrRiPsonSts_read/1 -> Terminator_443/1
  WtrActrRiResetSts_WtrActrRiResetSts_read/1 -> Terminator_444/1
  WtrActrRiSpclFct_WtrActrRiSpclFct_read/1 -> Terminator_445/1
  WtrActrRiSpdSts_WtrActrRiSpdSts_read/1 -> Terminator_446/1
  WtrActrRiTrqSts_WtrActrRiTrqSts_read/1 -> Terminator_447/1
  WtrActrRiUrgcExist_WtrActrRiUrgcExist_read/1 -> Terminator_448/1
  WtrActrRiUrgcPson_WtrActrRiUrgcPson_read/1 -> Terminator_449/1
  WtrActrRiUrgcSts_WtrActrRiUrgcSts_read/1 -> Terminator_450/1
  WtrActrRiVoltErr_WtrActrRiVoltErr_read/1 -> Terminator_451/1
  BswLifeCycle_LifeCycle_read/1 -> Terminator_452/1
  DioServ_PowerOffInitChannel/1 -> Terminator_453/1
  DioServ_PowerOnInitChannel/1 -> Terminator_454/1
  Ground_1/1 -> DioServ_ReadChannel/1
  DioServ_ReadChannel/1 -> Terminator_455/1
  DioServ_ReadChannel/2 -> Terminator_456/1
  Ground_2/1 -> DioServ_WriteChannel/1
  Ground_3/1 -> DioServ_WriteChannel/2
  DioServ_WriteChannel/1 -> Terminator_457/1
  Ground_4/1 -> LinIfServ_GetScheduleInfo/1
  LinIfServ_GetScheduleInfo/1 -> Terminator_458/1
  LinIfServ_GetScheduleInfo/2 -> Terminator_459/1
  Ground_5/1 -> LinSMServ_ScheduleRequest/1
  Ground_6/1 -> LinSMServ_ScheduleRequest/2
  LinSMServ_ScheduleRequest/1 -> Terminator_460/1
  LinService_GetLin2WakeupStatus/1 -> Terminator_461/1
  LinService_GetLin2WakeupStatus/2 -> Terminator_462/1
  Ground_7/1 -> LinService_SendLin3dFrame/1
  Ground_8/1 -> LinService_SendLin3dFrame/2
  LinService_SendLin3dFrame/1 -> Terminator_463/1
  Ground_9/1 -> LinService_SetLin3cFrame/1
  Ground_10/1 -> LinService_SetLin3cFrame/2
  LinService_SetLin3cFrame/1 -> Terminator_464/1
  Ground_11/1 -> LinService_SetLinTable/1
  Ground_12/1 -> LinService_SetLinTable/2
  LinService_SetLinTable/1 -> Terminator_465/1
  Ground_13/1 -> MirrorLin4ErrServ_GetHfaCcmLin4Fr01NoResp/1
  MirrorLin4ErrServ_GetHfaCcmLin4Fr01NoResp/1 -> Terminator_466/1
  MirrorLin4ErrServ_GetHfaCcmLin4Fr01NoResp/2 -> Terminator_467/1
  Ground_14/1 -> MirrorLin4ErrServ_GetHfaRxErrCurSts/1
  MirrorLin4ErrServ_GetHfaRxErrCurSts/1 -> Terminator_468/1
  MirrorLin4ErrServ_GetHfaRxErrCurSts/2 -> Terminator_469/1
  Ground_15/1 -> MirrorLin4ErrServ_GetHfaRxErrSta/1
  MirrorLin4ErrServ_GetHfaRxErrSta/1 -> Terminator_470/1
  MirrorLin4ErrServ_GetHfaRxErrSta/2 -> Terminator_471/1
  MirrorLin4ErrServ_GetLinBusTxErrSta/1 -> Terminator_472/1
  MirrorLin4ErrServ_GetLinBusTxErrSta/2 -> Terminator_473/1
  Ground_16/1 -> MirrorLinFrameServ_GetRespSta/1
  MirrorLinFrameServ_GetRespSta/1 -> Terminator_474/1
  MirrorLinFrameServ_GetRespSta/2 -> Terminator_475/1
  MirrorLinFrameServ_GetRespSta/3 -> Terminator_476/1
  NvMServ_HfaMtrCalReadBlock/1 -> Terminator_477/1
  NvMServ_HfaMtrCalReadBlock/2 -> Terminator_478/1
  Ground_17/1 -> NvMServ_HfaMtrCalWriteBlock/1
  NvMServ_HfaMtrCalWriteBlock/1 -> Terminator_479/1
  Ground_18/1 -> NvMServ_NvMNotifyJobWorking/1
  NvMServ_NvMNotifyJobWorking/1 -> Terminator_480/1
  NvMServ_NvMNotifyJobWorking/2 -> Terminator_481/1
  NvMServ_VgaMtrCalReadBlock/1 -> Terminator_482/1
  NvMServ_VgaMtrCalReadBlock/2 -> Terminator_483/1
  Ground_19/1 -> NvMServ_VgaMtrCalWriteBlock/1
  NvMServ_VgaMtrCalWriteBlock/1 -> Terminator_484/1
  Ground_20/1 -> NvMServ_WriteBlock/1
  Ground_21/1 -> NvMServ_WriteBlock/2
  NvMServ_WriteBlock/1 -> Terminator_485/1
  PA_NvMBlockHfaMtrCalDataInit/1 -> Terminator_486/1
  Ground_22/1 -> PwrParaService_GetMotorCailCnt/1
  PwrParaService_GetMotorCailCnt/1 -> Terminator_487/1
  PwrParaService_GetMotorCailCnt/2 -> Terminator_488/1
  Ground_23/1 -> PwrParaService_GetMotorCailNum/1
  PwrParaService_GetMotorCailNum/1 -> Terminator_489/1
  PwrParaService_GetMotorCailNum/2 -> Terminator_490/1
  Ground_24/1 -> PwrParaService_SetMotorCailCnt/1
  Ground_25/1 -> PwrParaService_SetMotorCailCnt/2
  PwrParaService_SetMotorCailCnt/1 -> Terminator_491/1
  Ground_26/1 -> PwrParaService_SetMotorCailNum/1
  Ground_27/1 -> PwrParaService_SetMotorCailNum/2
  PwrParaService_SetMotorCailNum/1 -> Terminator_492/1
  Ground_28/1 -> ActCfrAddrFinalAddr_ActCfrAddrFinalAddr_write/1
  Ground_29/1 -> ActCfrAddrGroup_ActCfrAddrGroup_write/1
  Ground_30/1 -> ActCfrAddrItem_ActCfrAddrItem_write/1
  Ground_31/1 -> ActCfrIniCPos_ActCfrIniCPos_write/1
  Ground_32/1 -> ActCfrIniGroup_ActCfrIniGroup_write/1
  Ground_33/1 -> ActCfrIniItem_ActCfrIniItem_write/1
  Ground_34/1 -> ActCfrIniResvd_ActCfrIniResvd_write/1
  Ground_35/1 -> ActCfrModGroup_ActCfrModGroup_write/1
  Ground_36/1 -> ActCfrModItem_ActCfrModItem_write/1
  Ground_37/1 -> ActCfrModNewMod_ActCfrModNewMod_write/1
  Ground_38/1 -> ActCfrModResvd1_ActCfrModResvd1_write/1
  Ground_39/1 -> ActCfrModResvd2_ActCfrModResvd2_write/1
  Ground_40/1 -> ActCfrPrgDiret_ActCfrPrgDiret_write/1
  Ground_41/1 -> ActCfrPrgGroup_ActCfrPrgGroup_write/1
  Ground_42/1 -> ActCfrPrgItem_ActCfrPrgItem_write/1
  Ground_43/1 -> ActCfrPrgPrgGroup_ActCfrPrgPrgGroup_write/1
  Ground_44/1 -> ActCfrPrgPrgItem_ActCfrPrgPrgItem_write/1
  Ground_45/1 -> ActCfrPrgRef_ActCfrPrgRef_write/1
  Ground_46/1 -> ActCfrPrgSafety_ActCfrPrgSafety_write/1
  Ground_47/1 -> ActCfrPrgSafetyEnable_ActCfrPrgSafetyEnable_write/1
  Ground_48/1 -> ActCfrSetAutoSpd_ActCfrSetAutoSpd_write/1
  Ground_49/1 -> ActCfrSetFPos_ActCfrSetFPos_write/1
  Ground_50/1 -> ActCfrSetGroup_ActCfrSetGroup_write/1
  Ground_51/1 -> ActCfrSetItem_ActCfrSetItem_write/1
  Ground_52/1 -> ActCfrSetLowNoise_ActCfrSetLowNoise_write/1
  Ground_53/1 -> ActCfrSetSpd_ActCfrSetSpd_write/1
  Ground_54/1 -> ActCfrSetStallDet_ActCfrSetStallDet_write/1
  Ground_55/1 -> ActCfrSetStopPhs_ActCfrSetStopPhs_write/1
  Ground_56/1 -> ActDfrIdeGroup_ActDfrIdeGroup_write/1
  Ground_57/1 -> ActDfrIdeItem_ActDfrIdeItem_write/1
  Ground_58/1 -> ActDfrIdeResvd_ActDfrIdeResvd_write/1
  Ground_59/1 -> ActDfrPrgGroup_ActDfrPrgGroup_write/1
  Ground_60/1 -> ActDfrPrgItem_ActDfrPrgItem_write/1
  Ground_61/1 -> ActDfrPrgResvd_ActDfrPrgResvd_write/1
  Ground_62/1 -> ActDfrStaGroup_ActDfrStaGroup_write/1
  Ground_63/1 -> ActDfrStaItem_ActDfrStaItem_write/1
  Ground_64/1 -> ActDfrStaResvd_ActDfrStaResvd_write/1
  Ground_65/1 -> CcmHfaMasterReqB0Lin4_CcmHfaMasterReqB0Lin4_write/1
  Ground_66/1 -> CcmHfaMasterReqB1Lin4_CcmHfaMasterReqB1Lin4_write/1
  Ground_67/1 -> CcmHfaMasterReqB2Lin4_CcmHfaMasterReqB2Lin4_write/1
  Ground_68/1 -> CcmHfaMasterReqB3Lin4_CcmHfaMasterReqB3Lin4_write/1
  Ground_69/1 -> CcmHfaMasterReqB4Lin4_CcmHfaMasterReqB3Lin4_write/1
  Ground_70/1 -> CcmHfaMasterReqB5Lin4_CcmHfaMasterReqB5Lin4_write/1
  Ground_71/1 -> CcmHfaMasterReqB6Lin4_CcmHfaMasterReqB6Lin4_write/1
  Ground_72/1 -> CcmHfaMasterReqB7Lin4_CcmHfaMasterReqB7Lin4_write/1
  Ground_73/1 -> Lin1HfaActvReq_Lin1HfaActvReq_write/1
  Ground_74/1 -> ReHvacMotAdr_ReHvacMotAdr_write/1
  Ground_75/1 -> ReHvacMotBlockCmd_ReHvacMotBlockCmd_write/1
  Ground_76/1 -> ReHvacMotClearReq_ReHvacMotClearReq_write/1
  Ground_77/1 -> ReHvacMotCoilPwrOn_ReHvacMotCoilPwrOn_write/1
  Ground_78/1 -> ReHvacMotDir_ReHvacMotDir_write/1
  Ground_79/1 -> ReHvacMotMode_ReHvacMotMode_write/1
  Ground_80/1 -> ReHvacMotPsonAvl_ReHvacMotPsonAvl_write/1
  Ground_81/1 -> ReHvacMotSaveData_ReHvacMotSaveData_write/1
  Ground_82/1 -> ReHvacMotSpdSet_ReHvacMotSpdSet_write/1
  Ground_83/1 -> ReHvacMotStrtPson_ReHvacMotStrtPson_write/1
  Ground_84/1 -> ReHvacMotTargtPson_ReHvacMotTargtPson_write/1
  Ground_85/1 -> ReHvacMotUrgcPson_ReHvacMotUrgcPson_write/1
  Ground_86/1 -> ReHvacMotUrgcUnlck_ReHvacMotUrgcUnlck_write/1
  Ground_87/1 -> RHFAScheTableReq_Val_write/1
  Ground_88/1 -> WtrMotAdr_WtrMotAdr_write/1
  Ground_89/1 -> WtrMotBlockCmd_WtrMotBlockCmd_write/1
  Ground_90/1 -> WtrMotClearReq_WtrMotClearReq_write/1
  Ground_91/1 -> WtrMotCoilPwrOn_WtrMotCoilPwrOn_write/1
  Ground_92/1 -> WtrMotDir_WtrMotDir_write/1
  Ground_93/1 -> WtrMotMode_WtrMotMode_write/1
  Ground_94/1 -> WtrMotPsonAvl_WtrMotPsonAvl_write/1
  Ground_95/1 -> WtrMotSaveData_WtrMotSaveData_write/1
  Ground_96/1 -> WtrMotSpdSet_WtrMotSpdSet_write/1
  Ground_97/1 -> WtrMotStrtPson_WtrMotStrtPson_write/1
  Ground_98/1 -> WtrMotTargtPson_WtrMotTargtPson_write/1
  Ground_99/1 -> WtrMotUrgcPson_WtrMotUrgcPson_write/1
  Ground_100/1 -> WtrMotUrgcUnlck_WtrMotUrgcUnlck_write/1
  Ground_101/1 -> HfaMotAdr_HfaMotAdr_write/1
  Ground_102/1 -> HfaMotBlockCmd_HfaMotBlockCmd_write/1
  Ground_103/1 -> HfaMotClearReq_HfaMotClearReq_write/1
  Ground_104/1 -> HfaMotCoilPwrOn_HfaMotCoilPwrOn_write/1
  Ground_105/1 -> HfaMotDir_HfaMotDir_write/1
  Ground_106/1 -> HfaMotMode_HfaMotMode_write/1
  Ground_107/1 -> HfaMotPsonAvl_HfaMotPsonAvl_write/1
  Ground_108/1 -> HfaMotSaveData_HfaMotSaveData_write/1
  Ground_109/1 -> HfaMotSpdSet_HfaMotSpdSet_write/1
  Ground_110/1 -> HfaMotStrtPson_HfaMotStrtPson_write/1
  Ground_111/1 -> HfaMotTargtPson_HfaMotTargtPson_write/1
  Ground_112/1 -> HfaMotUrgcPson_HfaMotUrgcPson_write/1
  Ground_113/1 -> HfaMotUrgcUnlck_HfaMotUrgcUnlck_write/1
